simulink model "os_polyphase_synth_4096c"  (R2021a) (continued)
== system os_polyphase_synth_4096c/pfb_fir/pol1_in1_tap85 == (continued)
      | PCIN+D*B+P+CARRYIN
      | PCIN+D*BCIN
      | PCIN+D*BCIN+C
      | PCIN+D*BCIN+C+CARRYIN
      | PCIN+D*BCIN+CARRYIN
      | PCIN+D*BCIN+P
      | PCIN+D*BCIN+P+CARRYIN
      | PCIN+D*D
      | PCIN+D*D+C
      | PCIN+D*D+C+CARRYIN
      | PCIN+D*D+CARRYIN
      | PCIN+D*D+P
      | PCIN+D*D+P+CARRYIN
      | PCIN+D+C
      | PCIN+D+C+CARRYIN
      | PCIN+D+CARRYIN
      | PCIN+D+P
      | PCIN+D+P+CARRYIN
      | PCIN+P
      | PCIN+P+(A+D)
      | PCIN+P+(A+D)*(A+D)
      | PCIN+P+(A+D)*(A+D)+CARRYIN
      | PCIN+P+(A+D)*B
      | PCIN+P+(A+D)*B+CARRYIN
      | PCIN+P+(A+D)*BCIN
      | PCIN+P+(A+D)*BCIN+CARRYIN
      | PCIN+P+(A+D)+CARRYIN
      | PCIN+P+(ACIN+D)
      | PCIN+P+(ACIN+D)*(ACIN+D)
      | PCIN+P+(ACIN+D)*(ACIN+D)+CARRYIN
      | PCIN+P+(ACIN+D)*B
      | PCIN+P+(ACIN+D)*B+CARRYIN
      | PCIN+P+(ACIN+D)*BCIN
      | PCIN+P+(ACIN+D)*BCIN+CARRYIN
      | PCIN+P+(ACIN+D)+CARRYIN
      | PCIN+P+(D+A)
      | PCIN+P+(D+A)*(D+A)
      | PCIN+P+(D+A)*(D+A)+CARRYIN
      | PCIN+P+(D+A)*B
      | PCIN+P+(D+A)*B+CARRYIN
      | PCIN+P+(D+A)*BCIN
      | PCIN+P+(D+A)*BCIN+CARRYIN
      | PCIN+P+(D+A)+CARRYIN
      | PCIN+P+(D+ACIN)
      | PCIN+P+(D+ACIN)*(D+ACIN)
      | PCIN+P+(D+ACIN)*(D+ACIN)+CARRYIN
      | PCIN+P+(D+ACIN)*B
      | PCIN+P+(D+ACIN)*B+CARRYIN
      | PCIN+P+(D+ACIN)*BCIN
      | PCIN+P+(D+ACIN)*BCIN+CARRYIN
      | PCIN+P+(D+ACIN)+CARRYIN
      | PCIN+P+(D-A)
      | PCIN+P+(D-A)*(D-A)
      | PCIN+P+(D-A)*(D-A)+CARRYIN
      | PCIN+P+(D-A)*B
      | PCIN+P+(D-A)*B+CARRYIN
      | PCIN+P+(D-A)*BCIN
      | PCIN+P+(D-A)*BCIN+CARRYIN
      | PCIN+P+(D-A)+CARRYIN
      | PCIN+P+(D-ACIN)
      | PCIN+P+(D-ACIN)*(D-ACIN)
      | PCIN+P+(D-ACIN)*(D-ACIN)+CARRYIN
      | PCIN+P+(D-ACIN)*B
      | PCIN+P+(D-ACIN)*B+CARRYIN
      | PCIN+P+(D-ACIN)*BCIN
      | PCIN+P+(D-ACIN)*BCIN+CARRYIN
      | PCIN+P+(D-ACIN)+CARRYIN
      | PCIN+P+A
      | PCIN+P+A*A
      | PCIN+P+A*A+CARRYIN
      | PCIN+P+A*B
      | PCIN+P+A*B+CARRYIN
      | PCIN+P+A*BCIN
      | PCIN+P+A*BCIN+CARRYIN
      | PCIN+P+A+CARRYIN
      | PCIN+P+ACIN
      | PCIN+P+ACIN*ACIN
      | PCIN+P+ACIN*ACIN+CARRYIN
      | PCIN+P+ACIN*B
      | PCIN+P+ACIN*B+CARRYIN
      | PCIN+P+ACIN*BCIN
      | PCIN+P+ACIN*BCIN+CARRYIN
      | PCIN+P+ACIN+CARRYIN
      | PCIN+P+B*(A+D)
      | PCIN+P+B*(A+D)+CARRYIN
      | PCIN+P+B*(ACIN+D)
      | PCIN+P+B*(ACIN+D)+CARRYIN
      | PCIN+P+B*(D+A)
      | PCIN+P+B*(D+A)+CARRYIN
      | PCIN+P+B*(D+ACIN)
      | PCIN+P+B*(D+ACIN)+CARRYIN
      | PCIN+P+B*(D-A)
      | PCIN+P+B*(D-A)+CARRYIN
      | PCIN+P+B*(D-ACIN)
      | PCIN+P+B*(D-ACIN)+CARRYIN
      | PCIN+P+B*A
      | PCIN+P+B*A+CARRYIN
      | PCIN+P+B*ACIN
      | PCIN+P+B*ACIN+CARRYIN
      | PCIN+P+B*D
      | PCIN+P+B*D+CARRYIN
      | PCIN+P+BCIN*(A+D)
      | PCIN+P+BCIN*(A+D)+CARRYIN
      | PCIN+P+BCIN*(ACIN+D)
      | PCIN+P+BCIN*(ACIN+D)+CARRYIN
      | PCIN+P+BCIN*(D+A)
      | PCIN+P+BCIN*(D+A)+CARRYIN
      | PCIN+P+BCIN*(D+ACIN)
      | PCIN+P+BCIN*(D+ACIN)+CARRYIN
      | PCIN+P+BCIN*(D-A)
      | PCIN+P+BCIN*(D-A)+CARRYIN
      | PCIN+P+BCIN*(D-ACIN)
      | PCIN+P+BCIN*(D-ACIN)+CARRYIN
      | PCIN+P+BCIN*A
      | PCIN+P+BCIN*A+CARRYIN
      | PCIN+P+BCIN*ACIN
      | PCIN+P+BCIN*ACIN+CARRYIN
      | PCIN+P+BCIN*D
      | PCIN+P+BCIN*D+CARRYIN
      | PCIN+P+C
      | PCIN+P+C+C
      | PCIN+P+C+C+CARRYIN
      | PCIN+P+C+CARRYIN
      | PCIN+P+C+CONCAT
      | PCIN+P+C+CONCAT+CARRYIN
      | PCIN+P+C+P
      | PCIN+P+C+P+CARRYIN
      | PCIN+P+CARRYIN
      | PCIN+P+CONCAT
      | PCIN+P+CONCAT+C
      | PCIN+P+CONCAT+C+CARRYIN
      | PCIN+P+CONCAT+CARRYIN
      | PCIN+P+D
      | PCIN+P+D*B
      | PCIN+P+D*B+CARRYIN
      | PCIN+P+D*BCIN
      | PCIN+P+D*BCIN+CARRYIN
      | PCIN+P+D*D
      | PCIN+P+D*D+CARRYIN
      | PCIN+P+D+CARRYIN
      | PCIN+P+P
      | PCIN+P+P+C
      | PCIN+P+P+C+CARRYIN
      | PCIN+P+P+CARRYIN
      | PCIN-((A+D))
      | PCIN-((A+D)*(A+D))
      | PCIN-((A+D)*(A+D)+C)
      | PCIN-((A+D)*(A+D)+C+CARRYIN)
      | PCIN-((A+D)*(A+D)+CARRYIN)
      | PCIN-((A+D)*(A+D)+P)
      | PCIN-((A+D)*(A+D)+P+CARRYIN)
      | PCIN-((A+D)*B)
      | PCIN-((A+D)*B+C)
      | PCIN-((A+D)*B+C+CARRYIN)
      | PCIN-((A+D)*B+CARRYIN)
      | PCIN-((A+D)*B+P)
      | PCIN-((A+D)*B+P+CARRYIN)
      | PCIN-((A+D)*BCIN)
      | PCIN-((A+D)*BCIN+C)
      | PCIN-((A+D)*BCIN+C+CARRYIN)
      | PCIN-((A+D)*BCIN+CARRYIN)
      | PCIN-((A+D)*BCIN+P)
      | PCIN-((A+D)*BCIN+P+CARRYIN)
      | PCIN-((A+D)+C)
      | PCIN-((A+D)+C+CARRYIN)
      | PCIN-((A+D)+CARRYIN)
      | PCIN-((A+D)+P)
      | PCIN-((A+D)+P+CARRYIN)
      | PCIN-((ACIN+D))
      | PCIN-((ACIN+D)*(ACIN+D))
      | PCIN-((ACIN+D)*(ACIN+D)+C)
      | PCIN-((ACIN+D)*(ACIN+D)+C+CARRYIN)
      | PCIN-((ACIN+D)*(ACIN+D)+CARRYIN)
      | PCIN-((ACIN+D)*(ACIN+D)+P)
      | PCIN-((ACIN+D)*(ACIN+D)+P+CARRYIN)
      | PCIN-((ACIN+D)*B)
      | PCIN-((ACIN+D)*B+C)
      | PCIN-((ACIN+D)*B+C+CARRYIN)
      | PCIN-((ACIN+D)*B+CARRYIN)
      | PCIN-((ACIN+D)*B+P)
      | PCIN-((ACIN+D)*B+P+CARRYIN)
      | PCIN-((ACIN+D)*BCIN)
      | PCIN-((ACIN+D)*BCIN+C)
      | PCIN-((ACIN+D)*BCIN+C+CARRYIN)
      | PCIN-((ACIN+D)*BCIN+CARRYIN)
      | PCIN-((ACIN+D)*BCIN+P)
      | PCIN-((ACIN+D)*BCIN+P+CARRYIN)
      | PCIN-((ACIN+D)+C)
      | PCIN-((ACIN+D)+C+CARRYIN)
      | PCIN-((ACIN+D)+CARRYIN)
      | PCIN-((ACIN+D)+P)
      | PCIN-((ACIN+D)+P+CARRYIN)
      | PCIN-((D+A))
      | PCIN-((D+A)*(D+A))
      | PCIN-((D+A)*(D+A)+C)
      | PCIN-((D+A)*(D+A)+C+CARRYIN)
      | PCIN-((D+A)*(D+A)+CARRYIN)
      | PCIN-((D+A)*(D+A)+P)
      | PCIN-((D+A)*(D+A)+P+CARRYIN)
      | PCIN-((D+A)*B)
      | PCIN-((D+A)*B+C)
      | PCIN-((D+A)*B+C+CARRYIN)
      | PCIN-((D+A)*B+CARRYIN)
      | PCIN-((D+A)*B+P)
      | PCIN-((D+A)*B+P+CARRYIN)
      | PCIN-((D+A)*BCIN)
      | PCIN-((D+A)*BCIN+C)
      | PCIN-((D+A)*BCIN+C+CARRYIN)
      | PCIN-((D+A)*BCIN+CARRYIN)
      | PCIN-((D+A)*BCIN+P)
      | PCIN-((D+A)*BCIN+P+CARRYIN)
      | PCIN-((D+A)+C)
      | PCIN-((D+A)+C+CARRYIN)
      | PCIN-((D+A)+CARRYIN)
      | PCIN-((D+A)+P)
      | PCIN-((D+A)+P+CARRYIN)
      | PCIN-((D+ACIN))
      | PCIN-((D+ACIN)*(D+ACIN))
      | PCIN-((D+ACIN)*(D+ACIN)+C)
      | PCIN-((D+ACIN)*(D+ACIN)+C+CARRYIN)
      | PCIN-((D+ACIN)*(D+ACIN)+CARRYIN)
      | PCIN-((D+ACIN)*(D+ACIN)+P)
      | PCIN-((D+ACIN)*(D+ACIN)+P+CARRYIN)
      | PCIN-((D+ACIN)*B)
      | PCIN-((D+ACIN)*B+C)
      | PCIN-((D+ACIN)*B+C+CARRYIN)
      | PCIN-((D+ACIN)*B+CARRYIN)
      | PCIN-((D+ACIN)*B+P)
      | PCIN-((D+ACIN)*B+P+CARRYIN)
      | PCIN-((D+ACIN)*BCIN)
      | PCIN-((D+ACIN)*BCIN+C)
      | PCIN-((D+ACIN)*BCIN+C+CARRYIN)
      | PCIN-((D+ACIN)*BCIN+CARRYIN)
      | PCIN-((D+ACIN)*BCIN+P)
      | PCIN-((D+ACIN)*BCIN+P+CARRYIN)
      | PCIN-((D+ACIN)+C)
      | PCIN-((D+ACIN)+C+CARRYIN)
      | PCIN-((D+ACIN)+CARRYIN)
      | PCIN-((D+ACIN)+P)
      | PCIN-((D+ACIN)+P+CARRYIN)
      | PCIN-((D-A))
      | PCIN-((D-A)*(D-A))
      | PCIN-((D-A)*(D-A)+C)
      | PCIN-((D-A)*(D-A)+C+CARRYIN)
      | PCIN-((D-A)*(D-A)+CARRYIN)
      | PCIN-((D-A)*(D-A)+P)
      | PCIN-((D-A)*(D-A)+P+CARRYIN)
      | PCIN-((D-A)*B)
      | PCIN-((D-A)*B+C)
      | PCIN-((D-A)*B+C+CARRYIN)
      | PCIN-((D-A)*B+CARRYIN)
      | PCIN-((D-A)*B+P)
      | PCIN-((D-A)*B+P+CARRYIN)
      | PCIN-((D-A)*BCIN)
      | PCIN-((D-A)*BCIN+C)
      | PCIN-((D-A)*BCIN+C+CARRYIN)
      | PCIN-((D-A)*BCIN+CARRYIN)
      | PCIN-((D-A)*BCIN+P)
      | PCIN-((D-A)*BCIN+P+CARRYIN)
      | PCIN-((D-A)+C)
      | PCIN-((D-A)+C+CARRYIN)
      | PCIN-((D-A)+CARRYIN)
      | PCIN-((D-A)+P)
      | PCIN-((D-A)+P+CARRYIN)
      | PCIN-((D-ACIN))
      | PCIN-((D-ACIN)*(D-ACIN))
      | PCIN-((D-ACIN)*(D-ACIN)+C)
      | PCIN-((D-ACIN)*(D-ACIN)+C+CARRYIN)
      | PCIN-((D-ACIN)*(D-ACIN)+CARRYIN)
      | PCIN-((D-ACIN)*(D-ACIN)+P)
      | PCIN-((D-ACIN)*(D-ACIN)+P+CARRYIN)
      | PCIN-((D-ACIN)*B)
      | PCIN-((D-ACIN)*B+C)
      | PCIN-((D-ACIN)*B+C+CARRYIN)
      | PCIN-((D-ACIN)*B+CARRYIN)
      | PCIN-((D-ACIN)*B+P)
      | PCIN-((D-ACIN)*B+P+CARRYIN)
      | PCIN-((D-ACIN)*BCIN)
      | PCIN-((D-ACIN)*BCIN+C)
      | PCIN-((D-ACIN)*BCIN+C+CARRYIN)
      | PCIN-((D-ACIN)*BCIN+CARRYIN)
      | PCIN-((D-ACIN)*BCIN+P)
      | PCIN-((D-ACIN)*BCIN+P+CARRYIN)
      | PCIN-((D-ACIN)+C)
      | PCIN-((D-ACIN)+C+CARRYIN)
      | PCIN-((D-ACIN)+CARRYIN)
      | PCIN-((D-ACIN)+P)
      | PCIN-((D-ACIN)+P+CARRYIN)
      | PCIN-(A)
      | PCIN-(A*A)
      | PCIN-(A*A+C)
      | PCIN-(A*A+C+CARRYIN)
      | PCIN-(A*A+CARRYIN)
      | PCIN-(A*A+P)
      | PCIN-(A*A+P+CARRYIN)
      | PCIN-(A*B)
      | PCIN-(A*B+C)
      | PCIN-(A*B+C+CARRYIN)
      | PCIN-(A*B+CARRYIN)
      | PCIN-(A*B+P)
      | PCIN-(A*B+P+CARRYIN)
      | PCIN-(A*BCIN)
      | PCIN-(A*BCIN+C)
      | PCIN-(A*BCIN+C+CARRYIN)
      | PCIN-(A*BCIN+CARRYIN)
      | PCIN-(A*BCIN+P)
      | PCIN-(A*BCIN+P+CARRYIN)
      | PCIN-(A+C)
      | PCIN-(A+C+CARRYIN)
      | PCIN-(A+CARRYIN)
      | PCIN-(A+D)
      | PCIN-(A+D)*(A+D)
      | PCIN-(A+D)*(A+D)-C
      | PCIN-(A+D)*(A+D)-C-CARRYIN
      | PCIN-(A+D)*(A+D)-CARRYIN
      | PCIN-(A+D)*(A+D)-P
      | PCIN-(A+D)*(A+D)-P-CARRYIN
      | PCIN-(A+D)*B
      | PCIN-(A+D)*B-C
      | PCIN-(A+D)*B-C-CARRYIN
      | PCIN-(A+D)*B-CARRYIN
      | PCIN-(A+D)*B-P
      | PCIN-(A+D)*B-P-CARRYIN
      | PCIN-(A+D)*BCIN
      | PCIN-(A+D)*BCIN-C
      | PCIN-(A+D)*BCIN-C-CARRYIN
      | PCIN-(A+D)*BCIN-CARRYIN
      | PCIN-(A+D)*BCIN-P
      | PCIN-(A+D)*BCIN-P-CARRYIN
      | PCIN-(A+D)-C
      | PCIN-(A+D)-C-CARRYIN
      | PCIN-(A+D)-CARRYIN
      | PCIN-(A+D)-P
      | PCIN-(A+D)-P-CARRYIN
      | PCIN-(A+P)
      | PCIN-(A+P+CARRYIN)
      | PCIN-(ACIN)
      | PCIN-(ACIN*ACIN)
      | PCIN-(ACIN*ACIN+C)
      | PCIN-(ACIN*ACIN+C+CARRYIN)
      | PCIN-(ACIN*ACIN+CARRYIN)
      | PCIN-(ACIN*ACIN+P)
      | PCIN-(ACIN*ACIN+P+CARRYIN)
      | PCIN-(ACIN*B)
      | PCIN-(ACIN*B+C)
      | PCIN-(ACIN*B+C+CARRYIN)
      | PCIN-(ACIN*B+CARRYIN)
      | PCIN-(ACIN*B+P)
      | PCIN-(ACIN*B+P+CARRYIN)
      | PCIN-(ACIN*BCIN)
      | PCIN-(ACIN*BCIN+C)
      | PCIN-(ACIN*BCIN+C+CARRYIN)
      | PCIN-(ACIN*BCIN+CARRYIN)
      | PCIN-(ACIN*BCIN+P)
      | PCIN-(ACIN*BCIN+P+CARRYIN)
      | PCIN-(ACIN+C)
      | PCIN-(ACIN+C+CARRYIN)
      | PCIN-(ACIN+CARRYIN)
      | PCIN-(ACIN+D)
      | PCIN-(ACIN+D)*(ACIN+D)
      | PCIN-(ACIN+D)*(ACIN+D)-C
      | PCIN-(ACIN+D)*(ACIN+D)-C-CARRYIN
      | PCIN-(ACIN+D)*(ACIN+D)-CARRYIN
      | PCIN-(ACIN+D)*(ACIN+D)-P
      | PCIN-(ACIN+D)*(ACIN+D)-P-CARRYIN
      | PCIN-(ACIN+D)*B
      | PCIN-(ACIN+D)*B-C
      | PCIN-(ACIN+D)*B-C-CARRYIN
      | PCIN-(ACIN+D)*B-CARRYIN
      | PCIN-(ACIN+D)*B-P
      | PCIN-(ACIN+D)*B-P-CARRYIN
      | PCIN-(ACIN+D)*BCIN
      | PCIN-(ACIN+D)*BCIN-C
      | PCIN-(ACIN+D)*BCIN-C-CARRYIN
      | PCIN-(ACIN+D)*BCIN-CARRYIN
      | PCIN-(ACIN+D)*BCIN-P
      | PCIN-(ACIN+D)*BCIN-P-CARRYIN
      | PCIN-(ACIN+D)-C
      | PCIN-(ACIN+D)-C-CARRYIN
      | PCIN-(ACIN+D)-CARRYIN
      | PCIN-(ACIN+D)-P
      | PCIN-(ACIN+D)-P-CARRYIN
      | PCIN-(ACIN+P)
      | PCIN-(ACIN+P+CARRYIN)
      | PCIN-(B*(A+D))
      | PCIN-(B*(A+D)+C)
      | PCIN-(B*(A+D)+C+CARRYIN)
      | PCIN-(B*(A+D)+CARRYIN)
      | PCIN-(B*(A+D)+P)
      | PCIN-(B*(A+D)+P+CARRYIN)
      | PCIN-(B*(ACIN+D))
      | PCIN-(B*(ACIN+D)+C)
      | PCIN-(B*(ACIN+D)+C+CARRYIN)
      | PCIN-(B*(ACIN+D)+CARRYIN)
      | PCIN-(B*(ACIN+D)+P)
      | PCIN-(B*(ACIN+D)+P+CARRYIN)
      | PCIN-(B*(D+A))
      | PCIN-(B*(D+A)+C)
      | PCIN-(B*(D+A)+C+CARRYIN)
      | PCIN-(B*(D+A)+CARRYIN)
      | PCIN-(B*(D+A)+P)
      | PCIN-(B*(D+A)+P+CARRYIN)
      | PCIN-(B*(D+ACIN))
      | PCIN-(B*(D+ACIN)+C)
      | PCIN-(B*(D+ACIN)+C+CARRYIN)
      | PCIN-(B*(D+ACIN)+CARRYIN)
      | PCIN-(B*(D+ACIN)+P)
      | PCIN-(B*(D+ACIN)+P+CARRYIN)
      | PCIN-(B*(D-A))
      | PCIN-(B*(D-A)+C)
      | PCIN-(B*(D-A)+C+CARRYIN)
      | PCIN-(B*(D-A)+CARRYIN)
      | PCIN-(B*(D-A)+P)
      | PCIN-(B*(D-A)+P+CARRYIN)
      | PCIN-(B*(D-ACIN))
      | PCIN-(B*(D-ACIN)+C)
      | PCIN-(B*(D-ACIN)+C+CARRYIN)
      | PCIN-(B*(D-ACIN)+CARRYIN)
      | PCIN-(B*(D-ACIN)+P)
      | PCIN-(B*(D-ACIN)+P+CARRYIN)
      | PCIN-(B*A)
      | PCIN-(B*A+C)
      | PCIN-(B*A+C+CARRYIN)
      | PCIN-(B*A+CARRYIN)
      | PCIN-(B*A+P)
      | PCIN-(B*A+P+CARRYIN)
      | PCIN-(B*ACIN)
      | PCIN-(B*ACIN+C)
      | PCIN-(B*ACIN+C+CARRYIN)
      | PCIN-(B*ACIN+CARRYIN)
      | PCIN-(B*ACIN+P)
      | PCIN-(B*ACIN+P+CARRYIN)
      | PCIN-(B*D)
      | PCIN-(B*D+C)
      | PCIN-(B*D+C+CARRYIN)
      | PCIN-(B*D+CARRYIN)
      | PCIN-(B*D+P)
      | PCIN-(B*D+P+CARRYIN)
      | PCIN-(BCIN*(A+D))
      | PCIN-(BCIN*(A+D)+C)
      | PCIN-(BCIN*(A+D)+C+CARRYIN)
      | PCIN-(BCIN*(A+D)+CARRYIN)
      | PCIN-(BCIN*(A+D)+P)
      | PCIN-(BCIN*(A+D)+P+CARRYIN)
      | PCIN-(BCIN*(ACIN+D))
      | PCIN-(BCIN*(ACIN+D)+C)
      | PCIN-(BCIN*(ACIN+D)+C+CARRYIN)
      | PCIN-(BCIN*(ACIN+D)+CARRYIN)
      | PCIN-(BCIN*(ACIN+D)+P)
      | PCIN-(BCIN*(ACIN+D)+P+CARRYIN)
      | PCIN-(BCIN*(D+A))
      | PCIN-(BCIN*(D+A)+C)
      | PCIN-(BCIN*(D+A)+C+CARRYIN)
      | PCIN-(BCIN*(D+A)+CARRYIN)
      | PCIN-(BCIN*(D+A)+P)
      | PCIN-(BCIN*(D+A)+P+CARRYIN)
      | PCIN-(BCIN*(D+ACIN))
      | PCIN-(BCIN*(D+ACIN)+C)
      | PCIN-(BCIN*(D+ACIN)+C+CARRYIN)
      | PCIN-(BCIN*(D+ACIN)+CARRYIN)
      | PCIN-(BCIN*(D+ACIN)+P)
      | PCIN-(BCIN*(D+ACIN)+P+CARRYIN)
      | PCIN-(BCIN*(D-A))
      | PCIN-(BCIN*(D-A)+C)
      | PCIN-(BCIN*(D-A)+C+CARRYIN)
      | PCIN-(BCIN*(D-A)+CARRYIN)
      | PCIN-(BCIN*(D-A)+P)
      | PCIN-(BCIN*(D-A)+P+CARRYIN)
      | PCIN-(BCIN*(D-ACIN))
      | PCIN-(BCIN*(D-ACIN)+C)
      | PCIN-(BCIN*(D-ACIN)+C+CARRYIN)
      | PCIN-(BCIN*(D-ACIN)+CARRYIN)
      | PCIN-(BCIN*(D-ACIN)+P)
      | PCIN-(BCIN*(D-ACIN)+P+CARRYIN)
      | PCIN-(BCIN*A)
      | PCIN-(BCIN*A+C)
      | PCIN-(BCIN*A+C+CARRYIN)
      | PCIN-(BCIN*A+CARRYIN)
      | PCIN-(BCIN*A+P)
      | PCIN-(BCIN*A+P+CARRYIN)
      | PCIN-(BCIN*ACIN)
      | PCIN-(BCIN*ACIN+C)
      | PCIN-(BCIN*ACIN+C+CARRYIN)
      | PCIN-(BCIN*ACIN+CARRYIN)
      | PCIN-(BCIN*ACIN+P)
      | PCIN-(BCIN*ACIN+P+CARRYIN)
      | PCIN-(BCIN*D)
      | PCIN-(BCIN*D+C)
      | PCIN-(BCIN*D+C+CARRYIN)
      | PCIN-(BCIN*D+CARRYIN)
      | PCIN-(BCIN*D+P)
      | PCIN-(BCIN*D+P+CARRYIN)
      | PCIN-(C)
      | PCIN-(C+(A+D))
      | PCIN-(C+(A+D)*(A+D))
      | PCIN-(C+(A+D)*(A+D)+CARRYIN)
      | PCIN-(C+(A+D)*B)
      | PCIN-(C+(A+D)*B+CARRYIN)
      | PCIN-(C+(A+D)*BCIN)
      | PCIN-(C+(A+D)*BCIN+CARRYIN)
      | PCIN-(C+(A+D)+CARRYIN)
      | PCIN-(C+(ACIN+D))
      | PCIN-(C+(ACIN+D)*(ACIN+D))
      | PCIN-(C+(ACIN+D)*(ACIN+D)+CARRYIN)
      | PCIN-(C+(ACIN+D)*B)
      | PCIN-(C+(ACIN+D)*B+CARRYIN)
      | PCIN-(C+(ACIN+D)*BCIN)
      | PCIN-(C+(ACIN+D)*BCIN+CARRYIN)
      | PCIN-(C+(ACIN+D)+CARRYIN)
      | PCIN-(C+(D+A))
      | PCIN-(C+(D+A)*(D+A))
      | PCIN-(C+(D+A)*(D+A)+CARRYIN)
      | PCIN-(C+(D+A)*B)
      | PCIN-(C+(D+A)*B+CARRYIN)
      | PCIN-(C+(D+A)*BCIN)
      | PCIN-(C+(D+A)*BCIN+CARRYIN)
      | PCIN-(C+(D+A)+CARRYIN)
      | PCIN-(C+(D+ACIN))
      | PCIN-(C+(D+ACIN)*(D+ACIN))
      | PCIN-(C+(D+ACIN)*(D+ACIN)+CARRYIN)
      | PCIN-(C+(D+ACIN)*B)
      | PCIN-(C+(D+ACIN)*B+CARRYIN)
      | PCIN-(C+(D+ACIN)*BCIN)
      | PCIN-(C+(D+ACIN)*BCIN+CARRYIN)
      | PCIN-(C+(D+ACIN)+CARRYIN)
      | PCIN-(C+(D-A))
      | PCIN-(C+(D-A)*(D-A))
      | PCIN-(C+(D-A)*(D-A)+CARRYIN)
      | PCIN-(C+(D-A)*B)
      | PCIN-(C+(D-A)*B+CARRYIN)
      | PCIN-(C+(D-A)*BCIN)
      | PCIN-(C+(D-A)*BCIN+CARRYIN)
      | PCIN-(C+(D-A)+CARRYIN)
      | PCIN-(C+(D-ACIN))
      | PCIN-(C+(D-ACIN)*(D-ACIN))
      | PCIN-(C+(D-ACIN)*(D-ACIN)+CARRYIN)
      | PCIN-(C+(D-ACIN)*B)
      | PCIN-(C+(D-ACIN)*B+CARRYIN)
      | PCIN-(C+(D-ACIN)*BCIN)
      | PCIN-(C+(D-ACIN)*BCIN+CARRYIN)
      | PCIN-(C+(D-ACIN)+CARRYIN)
      | PCIN-(C+A)
      | PCIN-(C+A*A)
      | PCIN-(C+A*A+CARRYIN)
      | PCIN-(C+A*B)
      | PCIN-(C+A*B+CARRYIN)
      | PCIN-(C+A*BCIN)
      | PCIN-(C+A*BCIN+CARRYIN)
      | PCIN-(C+A+CARRYIN)
      | PCIN-(C+ACIN)
      | PCIN-(C+ACIN*ACIN)
      | PCIN-(C+ACIN*ACIN+CARRYIN)
      | PCIN-(C+ACIN*B)
      | PCIN-(C+ACIN*B+CARRYIN)
      | PCIN-(C+ACIN*BCIN)
      | PCIN-(C+ACIN*BCIN+CARRYIN)
      | PCIN-(C+ACIN+CARRYIN)
      | PCIN-(C+B*(A+D))
      | PCIN-(C+B*(A+D)+CARRYIN)
      | PCIN-(C+B*(ACIN+D))
      | PCIN-(C+B*(ACIN+D)+CARRYIN)
      | PCIN-(C+B*(D+A))
      | PCIN-(C+B*(D+A)+CARRYIN)
      | PCIN-(C+B*(D+ACIN))
      | PCIN-(C+B*(D+ACIN)+CARRYIN)
      | PCIN-(C+B*(D-A))
      | PCIN-(C+B*(D-A)+CARRYIN)
      | PCIN-(C+B*(D-ACIN))
      | PCIN-(C+B*(D-ACIN)+CARRYIN)
      | PCIN-(C+B*A)
      | PCIN-(C+B*A+CARRYIN)
      | PCIN-(C+B*ACIN)
      | PCIN-(C+B*ACIN+CARRYIN)
      | PCIN-(C+B*D)
      | PCIN-(C+B*D+CARRYIN)
      | PCIN-(C+BCIN*(A+D))
      | PCIN-(C+BCIN*(A+D)+CARRYIN)
      | PCIN-(C+BCIN*(ACIN+D))
      | PCIN-(C+BCIN*(ACIN+D)+CARRYIN)
      | PCIN-(C+BCIN*(D+A))
      | PCIN-(C+BCIN*(D+A)+CARRYIN)
      | PCIN-(C+BCIN*(D+ACIN))
      | PCIN-(C+BCIN*(D+ACIN)+CARRYIN)
      | PCIN-(C+BCIN*(D-A))
      | PCIN-(C+BCIN*(D-A)+CARRYIN)
      | PCIN-(C+BCIN*(D-ACIN))
      | PCIN-(C+BCIN*(D-ACIN)+CARRYIN)
      | PCIN-(C+BCIN*A)
      | PCIN-(C+BCIN*A+CARRYIN)
      | PCIN-(C+BCIN*ACIN)
      | PCIN-(C+BCIN*ACIN+CARRYIN)
      | PCIN-(C+BCIN*D)
      | PCIN-(C+BCIN*D+CARRYIN)
      | PCIN-(C+C)
      | PCIN-(C+C+CARRYIN)
      | PCIN-(C+C+CONCAT)
      | PCIN-(C+C+CONCAT+CARRYIN)
      | PCIN-(C+C+P)
      | PCIN-(C+C+P+CARRYIN)
      | PCIN-(C+CARRYIN)
      | PCIN-(C+CONCAT)
      | PCIN-(C+CONCAT+C)
      | PCIN-(C+CONCAT+C+CARRYIN)
      | PCIN-(C+CONCAT+CARRYIN)
      | PCIN-(C+CONCAT+P)
      | PCIN-(C+CONCAT+P+CARRYIN)
      | PCIN-(C+D)
      | PCIN-(C+D*B)
      | PCIN-(C+D*B+CARRYIN)
      | PCIN-(C+D*BCIN)
      | PCIN-(C+D*BCIN+CARRYIN)
      | PCIN-(C+D*D)
      | PCIN-(C+D*D+CARRYIN)
      | PCIN-(C+D+CARRYIN)
      | PCIN-(C+P)
      | PCIN-(C+P+C)
      | PCIN-(C+P+C+CARRYIN)
      | PCIN-(C+P+CARRYIN)
      | PCIN-(C+P+CONCAT)
      | PCIN-(C+P+CONCAT+CARRYIN)
      | PCIN-(C+P+P)
      | PCIN-(C+P+P+CARRYIN)
      | PCIN-(CARRYIN)
      | PCIN-(CONCAT)
      | PCIN-(CONCAT+C)
      | PCIN-(CONCAT+C+C)
      | PCIN-(CONCAT+C+C+CARRYIN)
      | PCIN-(CONCAT+C+CARRYIN)
      | PCIN-(CONCAT+C+P)
      | PCIN-(CONCAT+C+P+CARRYIN)
      | PCIN-(CONCAT+CARRYIN)
      | PCIN-(CONCAT+P)
      | PCIN-(CONCAT+P+C)
      | PCIN-(CONCAT+P+C+CARRYIN)
      | PCIN-(CONCAT+P+CARRYIN)
      | PCIN-(D)
      | PCIN-(D*B)
      | PCIN-(D*B+C)
      | PCIN-(D*B+C+CARRYIN)
      | PCIN-(D*B+CARRYIN)
      | PCIN-(D*B+P)
      | PCIN-(D*B+P+CARRYIN)
      | PCIN-(D*BCIN)
      | PCIN-(D*BCIN+C)
      | PCIN-(D*BCIN+C+CARRYIN)
      | PCIN-(D*BCIN+CARRYIN)
      | PCIN-(D*BCIN+P)
      | PCIN-(D*BCIN+P+CARRYIN)
      | PCIN-(D*D)
      | PCIN-(D*D+C)
      | PCIN-(D*D+C+CARRYIN)
      | PCIN-(D*D+CARRYIN)
      | PCIN-(D*D+P)
      | PCIN-(D*D+P+CARRYIN)
      | PCIN-(D+A)
      | PCIN-(D+A)*(D+A)
      | PCIN-(D+A)*(D+A)-C
      | PCIN-(D+A)*(D+A)-C-CARRYIN
      | PCIN-(D+A)*(D+A)-CARRYIN
      | PCIN-(D+A)*(D+A)-P
      | PCIN-(D+A)*(D+A)-P-CARRYIN
      | PCIN-(D+A)*B
      | PCIN-(D+A)*B-C
      | PCIN-(D+A)*B-C-CARRYIN
      | PCIN-(D+A)*B-CARRYIN
      | PCIN-(D+A)*B-P
      | PCIN-(D+A)*B-P-CARRYIN
      | PCIN-(D+A)*BCIN
      | PCIN-(D+A)*BCIN-C
      | PCIN-(D+A)*BCIN-C-CARRYIN
      | PCIN-(D+A)*BCIN-CARRYIN
      | PCIN-(D+A)*BCIN-P
      | PCIN-(D+A)*BCIN-P-CARRYIN
      | PCIN-(D+A)-C
      | PCIN-(D+A)-C-CARRYIN
      | PCIN-(D+A)-CARRYIN
      | PCIN-(D+A)-P
      | PCIN-(D+A)-P-CARRYIN
      | PCIN-(D+ACIN)
      | PCIN-(D+ACIN)*(D+ACIN)
      | PCIN-(D+ACIN)*(D+ACIN)-C
      | PCIN-(D+ACIN)*(D+ACIN)-C-CARRYIN
      | PCIN-(D+ACIN)*(D+ACIN)-CARRYIN
      | PCIN-(D+ACIN)*(D+ACIN)-P
      | PCIN-(D+ACIN)*(D+ACIN)-P-CARRYIN
      | PCIN-(D+ACIN)*B
      | PCIN-(D+ACIN)*B-C
      | PCIN-(D+ACIN)*B-C-CARRYIN
      | PCIN-(D+ACIN)*B-CARRYIN
      | PCIN-(D+ACIN)*B-P
      | PCIN-(D+ACIN)*B-P-CARRYIN
      | PCIN-(D+ACIN)*BCIN
      | PCIN-(D+ACIN)*BCIN-C
      | PCIN-(D+ACIN)*BCIN-C-CARRYIN
      | PCIN-(D+ACIN)*BCIN-CARRYIN
      | PCIN-(D+ACIN)*BCIN-P
      | PCIN-(D+ACIN)*BCIN-P-CARRYIN
      | PCIN-(D+ACIN)-C
      | PCIN-(D+ACIN)-C-CARRYIN
      | PCIN-(D+ACIN)-CARRYIN
      | PCIN-(D+ACIN)-P
      | PCIN-(D+ACIN)-P-CARRYIN
      | PCIN-(D+C)
      | PCIN-(D+C+CARRYIN)
      | PCIN-(D+CARRYIN)
      | PCIN-(D+P)
      | PCIN-(D+P+CARRYIN)
      | PCIN-(D-A)
      | PCIN-(D-A)*(D-A)
      | PCIN-(D-A)*(D-A)-C
      | PCIN-(D-A)*(D-A)-C-CARRYIN
      | PCIN-(D-A)*(D-A)-CARRYIN
      | PCIN-(D-A)*(D-A)-P
      | PCIN-(D-A)*(D-A)-P-CARRYIN
      | PCIN-(D-A)*B
      | PCIN-(D-A)*B-C
      | PCIN-(D-A)*B-C-CARRYIN
      | PCIN-(D-A)*B-CARRYIN
      | PCIN-(D-A)*B-P
      | PCIN-(D-A)*B-P-CARRYIN
      | PCIN-(D-A)*BCIN
      | PCIN-(D-A)*BCIN-C
      | PCIN-(D-A)*BCIN-C-CARRYIN
      | PCIN-(D-A)*BCIN-CARRYIN
      | PCIN-(D-A)*BCIN-P
      | PCIN-(D-A)*BCIN-P-CARRYIN
      | PCIN-(D-A)-C
      | PCIN-(D-A)-C-CARRYIN
      | PCIN-(D-A)-CARRYIN
      | PCIN-(D-A)-P
      | PCIN-(D-A)-P-CARRYIN
      | PCIN-(D-ACIN)
      | PCIN-(D-ACIN)*(D-ACIN)
      | PCIN-(D-ACIN)*(D-ACIN)-C
      | PCIN-(D-ACIN)*(D-ACIN)-C-CARRYIN
      | PCIN-(D-ACIN)*(D-ACIN)-CARRYIN
      | PCIN-(D-ACIN)*(D-ACIN)-P
      | PCIN-(D-ACIN)*(D-ACIN)-P-CARRYIN
      | PCIN-(D-ACIN)*B
      | PCIN-(D-ACIN)*B-C
      | PCIN-(D-ACIN)*B-C-CARRYIN
      | PCIN-(D-ACIN)*B-CARRYIN
      | PCIN-(D-ACIN)*B-P
      | PCIN-(D-ACIN)*B-P-CARRYIN
      | PCIN-(D-ACIN)*BCIN
      | PCIN-(D-ACIN)*BCIN-C
      | PCIN-(D-ACIN)*BCIN-C-CARRYIN
      | PCIN-(D-ACIN)*BCIN-CARRYIN
      | PCIN-(D-ACIN)*BCIN-P
      | PCIN-(D-ACIN)*BCIN-P-CARRYIN
      | PCIN-(D-ACIN)-C
      | PCIN-(D-ACIN)-C-CARRYIN
      | PCIN-(D-ACIN)-CARRYIN
      | PCIN-(D-ACIN)-P
      | PCIN-(D-ACIN)-P-CARRYIN
      | PCIN-(P)
      | PCIN-(P+(A+D))
      | PCIN-(P+(A+D)*(A+D))
      | PCIN-(P+(A+D)*(A+D)+CARRYIN)
      | PCIN-(P+(A+D)*B)
      | PCIN-(P+(A+D)*B+CARRYIN)
      | PCIN-(P+(A+D)*BCIN)
      | PCIN-(P+(A+D)*BCIN+CARRYIN)
      | PCIN-(P+(A+D)+CARRYIN)
      | PCIN-(P+(ACIN+D))
      | PCIN-(P+(ACIN+D)*(ACIN+D))
      | PCIN-(P+(ACIN+D)*(ACIN+D)+CARRYIN)
      | PCIN-(P+(ACIN+D)*B)
      | PCIN-(P+(ACIN+D)*B+CARRYIN)
      | PCIN-(P+(ACIN+D)*BCIN)
      | PCIN-(P+(ACIN+D)*BCIN+CARRYIN)
      | PCIN-(P+(ACIN+D)+CARRYIN)
      | PCIN-(P+(D+A))
      | PCIN-(P+(D+A)*(D+A))
      | PCIN-(P+(D+A)*(D+A)+CARRYIN)
      | PCIN-(P+(D+A)*B)
      | PCIN-(P+(D+A)*B+CARRYIN)
      | PCIN-(P+(D+A)*BCIN)
      | PCIN-(P+(D+A)*BCIN+CARRYIN)
      | PCIN-(P+(D+A)+CARRYIN)
      | PCIN-(P+(D+ACIN))
      | PCIN-(P+(D+ACIN)*(D+ACIN))
      | PCIN-(P+(D+ACIN)*(D+ACIN)+CARRYIN)
      | PCIN-(P+(D+ACIN)*B)
      | PCIN-(P+(D+ACIN)*B+CARRYIN)
      | PCIN-(P+(D+ACIN)*BCIN)
      | PCIN-(P+(D+ACIN)*BCIN+CARRYIN)
      | PCIN-(P+(D+ACIN)+CARRYIN)
      | PCIN-(P+(D-A))
      | PCIN-(P+(D-A)*(D-A))
      | PCIN-(P+(D-A)*(D-A)+CARRYIN)
      | PCIN-(P+(D-A)*B)
      | PCIN-(P+(D-A)*B+CARRYIN)
      | PCIN-(P+(D-A)*BCIN)
      | PCIN-(P+(D-A)*BCIN+CARRYIN)
      | PCIN-(P+(D-A)+CARRYIN)
      | PCIN-(P+(D-ACIN))
      | PCIN-(P+(D-ACIN)*(D-ACIN))
      | PCIN-(P+(D-ACIN)*(D-ACIN)+CARRYIN)
      | PCIN-(P+(D-ACIN)*B)
      | PCIN-(P+(D-ACIN)*B+CARRYIN)
      | PCIN-(P+(D-ACIN)*BCIN)
      | PCIN-(P+(D-ACIN)*BCIN+CARRYIN)
      | PCIN-(P+(D-ACIN)+CARRYIN)
      | PCIN-(P+A)
      | PCIN-(P+A*A)
      | PCIN-(P+A*A+CARRYIN)
      | PCIN-(P+A*B)
      | PCIN-(P+A*B+CARRYIN)
      | PCIN-(P+A*BCIN)
      | PCIN-(P+A*BCIN+CARRYIN)
      | PCIN-(P+A+CARRYIN)
      | PCIN-(P+ACIN)
      | PCIN-(P+ACIN*ACIN)
      | PCIN-(P+ACIN*ACIN+CARRYIN)
      | PCIN-(P+ACIN*B)
      | PCIN-(P+ACIN*B+CARRYIN)
      | PCIN-(P+ACIN*BCIN)
      | PCIN-(P+ACIN*BCIN+CARRYIN)
      | PCIN-(P+ACIN+CARRYIN)
      | PCIN-(P+B*(A+D))
      | PCIN-(P+B*(A+D)+CARRYIN)
      | PCIN-(P+B*(ACIN+D))
      | PCIN-(P+B*(ACIN+D)+CARRYIN)
      | PCIN-(P+B*(D+A))
      | PCIN-(P+B*(D+A)+CARRYIN)
      | PCIN-(P+B*(D+ACIN))
      | PCIN-(P+B*(D+ACIN)+CARRYIN)
      | PCIN-(P+B*(D-A))
      | PCIN-(P+B*(D-A)+CARRYIN)
      | PCIN-(P+B*(D-ACIN))
      | PCIN-(P+B*(D-ACIN)+CARRYIN)
      | PCIN-(P+B*A)
      | PCIN-(P+B*A+CARRYIN)
      | PCIN-(P+B*ACIN)
      | PCIN-(P+B*ACIN+CARRYIN)
      | PCIN-(P+B*D)
      | PCIN-(P+B*D+CARRYIN)
      | PCIN-(P+BCIN*(A+D))
      | PCIN-(P+BCIN*(A+D)+CARRYIN)
      | PCIN-(P+BCIN*(ACIN+D))
      | PCIN-(P+BCIN*(ACIN+D)+CARRYIN)
      | PCIN-(P+BCIN*(D+A))
      | PCIN-(P+BCIN*(D+A)+CARRYIN)
      | PCIN-(P+BCIN*(D+ACIN))
      | PCIN-(P+BCIN*(D+ACIN)+CARRYIN)
      | PCIN-(P+BCIN*(D-A))
      | PCIN-(P+BCIN*(D-A)+CARRYIN)
      | PCIN-(P+BCIN*(D-ACIN))
      | PCIN-(P+BCIN*(D-ACIN)+CARRYIN)
      | PCIN-(P+BCIN*A)
      | PCIN-(P+BCIN*A+CARRYIN)
      | PCIN-(P+BCIN*ACIN)
      | PCIN-(P+BCIN*ACIN+CARRYIN)
      | PCIN-(P+BCIN*D)
      | PCIN-(P+BCIN*D+CARRYIN)
      | PCIN-(P+C)
      | PCIN-(P+C+C)
      | PCIN-(P+C+C+CARRYIN)
      | PCIN-(P+C+CARRYIN)
      | PCIN-(P+C+CONCAT)
      | PCIN-(P+C+CONCAT+CARRYIN)
      | PCIN-(P+C+P)
      | PCIN-(P+C+P+CARRYIN)
      | PCIN-(P+CARRYIN)
      | PCIN-(P+CONCAT)
      | PCIN-(P+CONCAT+C)
      | PCIN-(P+CONCAT+C+CARRYIN)
      | PCIN-(P+CONCAT+CARRYIN)
      | PCIN-(P+D)
      | PCIN-(P+D*B)
      | PCIN-(P+D*B+CARRYIN)
      | PCIN-(P+D*BCIN)
      | PCIN-(P+D*BCIN+CARRYIN)
      | PCIN-(P+D*D)
      | PCIN-(P+D*D+CARRYIN)
      | PCIN-(P+D+CARRYIN)
      | PCIN-(P+P)
      | PCIN-(P+P+C)
      | PCIN-(P+P+C+CARRYIN)
      | PCIN-(P+P+CARRYIN)
      | PCIN-A
      | PCIN-A*A
      | PCIN-A*A-C
      | PCIN-A*A-C-CARRYIN
      | PCIN-A*A-CARRYIN
      | PCIN-A*A-P
      | PCIN-A*A-P-CARRYIN
      | PCIN-A*B
      | PCIN-A*B-C
      | PCIN-A*B-C-CARRYIN
      | PCIN-A*B-CARRYIN
      | PCIN-A*B-P
      | PCIN-A*B-P-CARRYIN
      | PCIN-A*BCIN
      | PCIN-A*BCIN-C
      | PCIN-A*BCIN-C-CARRYIN
      | PCIN-A*BCIN-CARRYIN
      | PCIN-A*BCIN-P
      | PCIN-A*BCIN-P-CARRYIN
      | PCIN-A-C
      | PCIN-A-C-CARRYIN
      | PCIN-A-CARRYIN
      | PCIN-A-P
      | PCIN-A-P-CARRYIN
      | PCIN-ACIN
      | PCIN-ACIN*ACIN
      | PCIN-ACIN*ACIN-C
      | PCIN-ACIN*ACIN-C-CARRYIN
      | PCIN-ACIN*ACIN-CARRYIN
      | PCIN-ACIN*ACIN-P
      | PCIN-ACIN*ACIN-P-CARRYIN
      | PCIN-ACIN*B
      | PCIN-ACIN*B-C
      | PCIN-ACIN*B-C-CARRYIN
      | PCIN-ACIN*B-CARRYIN
      | PCIN-ACIN*B-P
      | PCIN-ACIN*B-P-CARRYIN
      | PCIN-ACIN*BCIN
      | PCIN-ACIN*BCIN-C
      | PCIN-ACIN*BCIN-C-CARRYIN
      | PCIN-ACIN*BCIN-CARRYIN
      | PCIN-ACIN*BCIN-P
      | PCIN-ACIN*BCIN-P-CARRYIN
      | PCIN-ACIN-C
      | PCIN-ACIN-C-CARRYIN
      | PCIN-ACIN-CARRYIN
      | PCIN-ACIN-P
      | PCIN-ACIN-P-CARRYIN
      | PCIN-B*(A+D)
      | PCIN-B*(A+D)-C
      | PCIN-B*(A+D)-C-CARRYIN
      | PCIN-B*(A+D)-CARRYIN
      | PCIN-B*(A+D)-P
      | PCIN-B*(A+D)-P-CARRYIN
      | PCIN-B*(ACIN+D)
      | PCIN-B*(ACIN+D)-C
      | PCIN-B*(ACIN+D)-C-CARRYIN
      | PCIN-B*(ACIN+D)-CARRYIN
      | PCIN-B*(ACIN+D)-P
      | PCIN-B*(ACIN+D)-P-CARRYIN
      | PCIN-B*(D+A)
      | PCIN-B*(D+A)-C
      | PCIN-B*(D+A)-C-CARRYIN
      | PCIN-B*(D+A)-CARRYIN
      | PCIN-B*(D+A)-P
      | PCIN-B*(D+A)-P-CARRYIN
      | PCIN-B*(D+ACIN)
      | PCIN-B*(D+ACIN)-C
      | PCIN-B*(D+ACIN)-C-CARRYIN
      | PCIN-B*(D+ACIN)-CARRYIN
      | PCIN-B*(D+ACIN)-P
      | PCIN-B*(D+ACIN)-P-CARRYIN
      | PCIN-B*(D-A)
      | PCIN-B*(D-A)-C
      | PCIN-B*(D-A)-C-CARRYIN
      | PCIN-B*(D-A)-CARRYIN
      | PCIN-B*(D-A)-P
      | PCIN-B*(D-A)-P-CARRYIN
      | PCIN-B*(D-ACIN)
      | PCIN-B*(D-ACIN)-C
      | PCIN-B*(D-ACIN)-C-CARRYIN
      | PCIN-B*(D-ACIN)-CARRYIN
      | PCIN-B*(D-ACIN)-P
      | PCIN-B*(D-ACIN)-P-CARRYIN
      | PCIN-B*A
      | PCIN-B*A-C
      | PCIN-B*A-C-CARRYIN
      | PCIN-B*A-CARRYIN
      | PCIN-B*A-P
      | PCIN-B*A-P-CARRYIN
      | PCIN-B*ACIN
      | PCIN-B*ACIN-C
      | PCIN-B*ACIN-C-CARRYIN
      | PCIN-B*ACIN-CARRYIN
      | PCIN-B*ACIN-P
      | PCIN-B*ACIN-P-CARRYIN
      | PCIN-B*D
      | PCIN-B*D-C
      | PCIN-B*D-C-CARRYIN
      | PCIN-B*D-CARRYIN
      | PCIN-B*D-P
      | PCIN-B*D-P-CARRYIN
      | PCIN-BCIN*(A+D)
      | PCIN-BCIN*(A+D)-C
      | PCIN-BCIN*(A+D)-C-CARRYIN
      | PCIN-BCIN*(A+D)-CARRYIN
      | PCIN-BCIN*(A+D)-P
      | PCIN-BCIN*(A+D)-P-CARRYIN
      | PCIN-BCIN*(ACIN+D)
      | PCIN-BCIN*(ACIN+D)-C
      | PCIN-BCIN*(ACIN+D)-C-CARRYIN
      | PCIN-BCIN*(ACIN+D)-CARRYIN
      | PCIN-BCIN*(ACIN+D)-P
      | PCIN-BCIN*(ACIN+D)-P-CARRYIN
      | PCIN-BCIN*(D+A)
      | PCIN-BCIN*(D+A)-C
      | PCIN-BCIN*(D+A)-C-CARRYIN
      | PCIN-BCIN*(D+A)-CARRYIN
      | PCIN-BCIN*(D+A)-P
      | PCIN-BCIN*(D+A)-P-CARRYIN
      | PCIN-BCIN*(D+ACIN)
      | PCIN-BCIN*(D+ACIN)-C
      | PCIN-BCIN*(D+ACIN)-C-CARRYIN
      | PCIN-BCIN*(D+ACIN)-CARRYIN
      | PCIN-BCIN*(D+ACIN)-P
      | PCIN-BCIN*(D+ACIN)-P-CARRYIN
      | PCIN-BCIN*(D-A)
      | PCIN-BCIN*(D-A)-C
      | PCIN-BCIN*(D-A)-C-CARRYIN
      | PCIN-BCIN*(D-A)-CARRYIN
      | PCIN-BCIN*(D-A)-P
      | PCIN-BCIN*(D-A)-P-CARRYIN
      | PCIN-BCIN*(D-ACIN)
      | PCIN-BCIN*(D-ACIN)-C
      | PCIN-BCIN*(D-ACIN)-C-CARRYIN
      | PCIN-BCIN*(D-ACIN)-CARRYIN
      | PCIN-BCIN*(D-ACIN)-P
      | PCIN-BCIN*(D-ACIN)-P-CARRYIN
      | PCIN-BCIN*A
      | PCIN-BCIN*A-C
      | PCIN-BCIN*A-C-CARRYIN
      | PCIN-BCIN*A-CARRYIN
      | PCIN-BCIN*A-P
      | PCIN-BCIN*A-P-CARRYIN
      | PCIN-BCIN*ACIN
      | PCIN-BCIN*ACIN-C
      | PCIN-BCIN*ACIN-C-CARRYIN
      | PCIN-BCIN*ACIN-CARRYIN
      | PCIN-BCIN*ACIN-P
      | PCIN-BCIN*ACIN-P-CARRYIN
      | PCIN-BCIN*D
      | PCIN-BCIN*D-C
      | PCIN-BCIN*D-C-CARRYIN
      | PCIN-BCIN*D-CARRYIN
      | PCIN-BCIN*D-P
      | PCIN-BCIN*D-P-CARRYIN
      | PCIN-C
      | PCIN-C-(A+D)
      | PCIN-C-(A+D)*(A+D)
      | PCIN-C-(A+D)*(A+D)-CARRYIN
      | PCIN-C-(A+D)*B
      | PCIN-C-(A+D)*B-CARRYIN
      | PCIN-C-(A+D)*BCIN
      | PCIN-C-(A+D)*BCIN-CARRYIN
      | PCIN-C-(A+D)-CARRYIN
      | PCIN-C-(ACIN+D)
      | PCIN-C-(ACIN+D)*(ACIN+D)
      | PCIN-C-(ACIN+D)*(ACIN+D)-CARRYIN
      | PCIN-C-(ACIN+D)*B
      | PCIN-C-(ACIN+D)*B-CARRYIN
      | PCIN-C-(ACIN+D)*BCIN
      | PCIN-C-(ACIN+D)*BCIN-CARRYIN
      | PCIN-C-(ACIN+D)-CARRYIN
      | PCIN-C-(D+A)
      | PCIN-C-(D+A)*(D+A)
      | PCIN-C-(D+A)*(D+A)-CARRYIN
      | PCIN-C-(D+A)*B
      | PCIN-C-(D+A)*B-CARRYIN
      | PCIN-C-(D+A)*BCIN
      | PCIN-C-(D+A)*BCIN-CARRYIN
      | PCIN-C-(D+A)-CARRYIN
      | PCIN-C-(D+ACIN)
      | PCIN-C-(D+ACIN)*(D+ACIN)
      | PCIN-C-(D+ACIN)*(D+ACIN)-CARRYIN
      | PCIN-C-(D+ACIN)*B
      | PCIN-C-(D+ACIN)*B-CARRYIN
      | PCIN-C-(D+ACIN)*BCIN
      | PCIN-C-(D+ACIN)*BCIN-CARRYIN
      | PCIN-C-(D+ACIN)-CARRYIN
      | PCIN-C-(D-A)
      | PCIN-C-(D-A)*(D-A)
      | PCIN-C-(D-A)*(D-A)-CARRYIN
      | PCIN-C-(D-A)*B
      | PCIN-C-(D-A)*B-CARRYIN
      | PCIN-C-(D-A)*BCIN
      | PCIN-C-(D-A)*BCIN-CARRYIN
      | PCIN-C-(D-A)-CARRYIN
      | PCIN-C-(D-ACIN)
      | PCIN-C-(D-ACIN)*(D-ACIN)
      | PCIN-C-(D-ACIN)*(D-ACIN)-CARRYIN
      | PCIN-C-(D-ACIN)*B
      | PCIN-C-(D-ACIN)*B-CARRYIN
      | PCIN-C-(D-ACIN)*BCIN
      | PCIN-C-(D-ACIN)*BCIN-CARRYIN
      | PCIN-C-(D-ACIN)-CARRYIN
      | PCIN-C-A
      | PCIN-C-A*A
      | PCIN-C-A*A-CARRYIN
      | PCIN-C-A*B
      | PCIN-C-A*B-CARRYIN
      | PCIN-C-A*BCIN
      | PCIN-C-A*BCIN-CARRYIN
      | PCIN-C-A-CARRYIN
      | PCIN-C-ACIN
      | PCIN-C-ACIN*ACIN
      | PCIN-C-ACIN*ACIN-CARRYIN
      | PCIN-C-ACIN*B
      | PCIN-C-ACIN*B-CARRYIN
      | PCIN-C-ACIN*BCIN
      | PCIN-C-ACIN*BCIN-CARRYIN
      | PCIN-C-ACIN-CARRYIN
      | PCIN-C-B*(A+D)
      | PCIN-C-B*(A+D)-CARRYIN
      | PCIN-C-B*(ACIN+D)
      | PCIN-C-B*(ACIN+D)-CARRYIN
      | PCIN-C-B*(D+A)
      | PCIN-C-B*(D+A)-CARRYIN
      | PCIN-C-B*(D+ACIN)
      | PCIN-C-B*(D+ACIN)-CARRYIN
      | PCIN-C-B*(D-A)
      | PCIN-C-B*(D-A)-CARRYIN
      | PCIN-C-B*(D-ACIN)
      | PCIN-C-B*(D-ACIN)-CARRYIN
      | PCIN-C-B*A
      | PCIN-C-B*A-CARRYIN
      | PCIN-C-B*ACIN
      | PCIN-C-B*ACIN-CARRYIN
      | PCIN-C-B*D
      | PCIN-C-B*D-CARRYIN
      | PCIN-C-BCIN*(A+D)
      | PCIN-C-BCIN*(A+D)-CARRYIN
      | PCIN-C-BCIN*(ACIN+D)
      | PCIN-C-BCIN*(ACIN+D)-CARRYIN
      | PCIN-C-BCIN*(D+A)
      | PCIN-C-BCIN*(D+A)-CARRYIN
      | PCIN-C-BCIN*(D+ACIN)
      | PCIN-C-BCIN*(D+ACIN)-CARRYIN
      | PCIN-C-BCIN*(D-A)
      | PCIN-C-BCIN*(D-A)-CARRYIN
      | PCIN-C-BCIN*(D-ACIN)
      | PCIN-C-BCIN*(D-ACIN)-CARRYIN
      | PCIN-C-BCIN*A
      | PCIN-C-BCIN*A-CARRYIN
      | PCIN-C-BCIN*ACIN
      | PCIN-C-BCIN*ACIN-CARRYIN
      | PCIN-C-BCIN*D
      | PCIN-C-BCIN*D-CARRYIN
      | PCIN-C-C
      | PCIN-C-C-CARRYIN
      | PCIN-C-C-CONCAT
      | PCIN-C-C-CONCAT-CARRYIN
      | PCIN-C-C-P
      | PCIN-C-C-P-CARRYIN
      | PCIN-C-CARRYIN
      | PCIN-C-CONCAT
      | PCIN-C-CONCAT-C
      | PCIN-C-CONCAT-C-CARRYIN
      | PCIN-C-CONCAT-CARRYIN
      | PCIN-C-CONCAT-P
      | PCIN-C-CONCAT-P-CARRYIN
      | PCIN-C-D
      | PCIN-C-D*B
      | PCIN-C-D*B-CARRYIN
      | PCIN-C-D*BCIN
      | PCIN-C-D*BCIN-CARRYIN
      | PCIN-C-D*D
      | PCIN-C-D*D-CARRYIN
      | PCIN-C-D-CARRYIN
      | PCIN-C-P
      | PCIN-C-P-C
      | PCIN-C-P-C-CARRYIN
      | PCIN-C-P-CARRYIN
      | PCIN-C-P-CONCAT
      | PCIN-C-P-CONCAT-CARRYIN
      | PCIN-C-P-P
      | PCIN-C-P-P-CARRYIN
      | PCIN-CARRYIN
      | PCIN-CONCAT
      | PCIN-CONCAT-C
      | PCIN-CONCAT-C-C
      | PCIN-CONCAT-C-C-CARRYIN
      | PCIN-CONCAT-C-CARRYIN
      | PCIN-CONCAT-C-P
      | PCIN-CONCAT-C-P-CARRYIN
      | PCIN-CONCAT-CARRYIN
      | PCIN-CONCAT-P
      | PCIN-CONCAT-P-C
      | PCIN-CONCAT-P-C-CARRYIN
      | PCIN-CONCAT-P-CARRYIN
      | PCIN-D
      | PCIN-D*B
      | PCIN-D*B-C
      | PCIN-D*B-C-CARRYIN
      | PCIN-D*B-CARRYIN
      | PCIN-D*B-P
      | PCIN-D*B-P-CARRYIN
      | PCIN-D*BCIN
      | PCIN-D*BCIN-C
      | PCIN-D*BCIN-C-CARRYIN
      | PCIN-D*BCIN-CARRYIN
      | PCIN-D*BCIN-P
      | PCIN-D*BCIN-P-CARRYIN
      | PCIN-D*D
      | PCIN-D*D-C
      | PCIN-D*D-C-CARRYIN
      | PCIN-D*D-CARRYIN
      | PCIN-D*D-P
      | PCIN-D*D-P-CARRYIN
      | PCIN-D-C
      | PCIN-D-C-CARRYIN
      | PCIN-D-CARRYIN
      | PCIN-D-P
      | PCIN-D-P-CARRYIN
      | PCIN-P
      | PCIN-P-(A+D)
      | PCIN-P-(A+D)*(A+D)
      | PCIN-P-(A+D)*(A+D)-CARRYIN
      | PCIN-P-(A+D)*B
      | PCIN-P-(A+D)*B-CARRYIN
      | PCIN-P-(A+D)*BCIN
      | PCIN-P-(A+D)*BCIN-CARRYIN
      | PCIN-P-(A+D)-CARRYIN
      | PCIN-P-(ACIN+D)
      | PCIN-P-(ACIN+D)*(ACIN+D)
      | PCIN-P-(ACIN+D)*(ACIN+D)-CARRYIN
      | PCIN-P-(ACIN+D)*B
      | PCIN-P-(ACIN+D)*B-CARRYIN
      | PCIN-P-(ACIN+D)*BCIN
      | PCIN-P-(ACIN+D)*BCIN-CARRYIN
      | PCIN-P-(ACIN+D)-CARRYIN
      | PCIN-P-(D+A)
      | PCIN-P-(D+A)*(D+A)
      | PCIN-P-(D+A)*(D+A)-CARRYIN
      | PCIN-P-(D+A)*B
      | PCIN-P-(D+A)*B-CARRYIN
      | PCIN-P-(D+A)*BCIN
      | PCIN-P-(D+A)*BCIN-CARRYIN
      | PCIN-P-(D+A)-CARRYIN
      | PCIN-P-(D+ACIN)
      | PCIN-P-(D+ACIN)*(D+ACIN)
      | PCIN-P-(D+ACIN)*(D+ACIN)-CARRYIN
      | PCIN-P-(D+ACIN)*B
      | PCIN-P-(D+ACIN)*B-CARRYIN
      | PCIN-P-(D+ACIN)*BCIN
      | PCIN-P-(D+ACIN)*BCIN-CARRYIN
      | PCIN-P-(D+ACIN)-CARRYIN
      | PCIN-P-(D-A)
      | PCIN-P-(D-A)*(D-A)
      | PCIN-P-(D-A)*(D-A)-CARRYIN
      | PCIN-P-(D-A)*B
      | PCIN-P-(D-A)*B-CARRYIN
      | PCIN-P-(D-A)*BCIN
      | PCIN-P-(D-A)*BCIN-CARRYIN
      | PCIN-P-(D-A)-CARRYIN
      | PCIN-P-(D-ACIN)
      | PCIN-P-(D-ACIN)*(D-ACIN)
      | PCIN-P-(D-ACIN)*(D-ACIN)-CARRYIN
      | PCIN-P-(D-ACIN)*B
      | PCIN-P-(D-ACIN)*B-CARRYIN
      | PCIN-P-(D-ACIN)*BCIN
      | PCIN-P-(D-ACIN)*BCIN-CARRYIN
      | PCIN-P-(D-ACIN)-CARRYIN
      | PCIN-P-A
      | PCIN-P-A*A
      | PCIN-P-A*A-CARRYIN
      | PCIN-P-A*B
      | PCIN-P-A*B-CARRYIN
      | PCIN-P-A*BCIN
      | PCIN-P-A*BCIN-CARRYIN
      | PCIN-P-A-CARRYIN
      | PCIN-P-ACIN
      | PCIN-P-ACIN*ACIN
      | PCIN-P-ACIN*ACIN-CARRYIN
      | PCIN-P-ACIN*B
      | PCIN-P-ACIN*B-CARRYIN
      | PCIN-P-ACIN*BCIN
      | PCIN-P-ACIN*BCIN-CARRYIN
      | PCIN-P-ACIN-CARRYIN
      | PCIN-P-B*(A+D)
      | PCIN-P-B*(A+D)-CARRYIN
      | PCIN-P-B*(ACIN+D)
      | PCIN-P-B*(ACIN+D)-CARRYIN
      | PCIN-P-B*(D+A)
      | PCIN-P-B*(D+A)-CARRYIN
      | PCIN-P-B*(D+ACIN)
      | PCIN-P-B*(D+ACIN)-CARRYIN
      | PCIN-P-B*(D-A)
      | PCIN-P-B*(D-A)-CARRYIN
      | PCIN-P-B*(D-ACIN)
      | PCIN-P-B*(D-ACIN)-CARRYIN
      | PCIN-P-B*A
      | PCIN-P-B*A-CARRYIN
      | PCIN-P-B*ACIN
      | PCIN-P-B*ACIN-CARRYIN
      | PCIN-P-B*D
      | PCIN-P-B*D-CARRYIN
      | PCIN-P-BCIN*(A+D)
      | PCIN-P-BCIN*(A+D)-CARRYIN
      | PCIN-P-BCIN*(ACIN+D)
      | PCIN-P-BCIN*(ACIN+D)-CARRYIN
      | PCIN-P-BCIN*(D+A)
      | PCIN-P-BCIN*(D+A)-CARRYIN
      | PCIN-P-BCIN*(D+ACIN)
      | PCIN-P-BCIN*(D+ACIN)-CARRYIN
      | PCIN-P-BCIN*(D-A)
      | PCIN-P-BCIN*(D-A)-CARRYIN
      | PCIN-P-BCIN*(D-ACIN)
      | PCIN-P-BCIN*(D-ACIN)-CARRYIN
      | PCIN-P-BCIN*A
      | PCIN-P-BCIN*A-CARRYIN
      | PCIN-P-BCIN*ACIN
      | PCIN-P-BCIN*ACIN-CARRYIN
      | PCIN-P-BCIN*D
      | PCIN-P-BCIN*D-CARRYIN
      | PCIN-P-C
      | PCIN-P-C-C
      | PCIN-P-C-C-CARRYIN
      | PCIN-P-C-CARRYIN
      | PCIN-P-C-CONCAT
      | PCIN-P-C-CONCAT-CARRYIN
      | PCIN-P-C-P
      | PCIN-P-C-P-CARRYIN
      | PCIN-P-CARRYIN
      | PCIN-P-CONCAT
      | PCIN-P-CONCAT-C
      | PCIN-P-CONCAT-C-CARRYIN
      | PCIN-P-CONCAT-CARRYIN
      | PCIN-P-D
      | PCIN-P-D*B
      | PCIN-P-D*B-CARRYIN
      | PCIN-P-D*BCIN
      | PCIN-P-D*BCIN-CARRYIN
      | PCIN-P-D*D
      | PCIN-P-D*D-CARRYIN
      | PCIN-P-D-CARRYIN
      | PCIN-P-P
      | PCIN-P-P-C
      | PCIN-P-P-C-CARRYIN
      | PCIN-P-P-CARRYIN
      | PCIN>>17
      | PCIN>>17+(A+D)
      | PCIN>>17+(A+D)*(A+D)
      | PCIN>>17+(A+D)*(A+D)+C